simulink model "chapter2_5_2"  (R2022a) (continued)
== system chapter2_5_2/Subsystem5/Subsystem3/Subsystem6 == (continued)
  Add Constant9/1 -> Subsystem11/1(index), Add Constant10/1
  Add Constant3/1 -> Subsystem6/1(index), Add Constant4/1
  Add Constant13/1 -> Add Constant19/1, Subsystem16/1(index)
  Subsystem16/1(Out1) -> Add/18
  [Ff] -> Subsystem19/2(F)

== system chapter2_5_2/Subsystem5/Subsystem3/Subsystem7 ==
  In2 : Inport
  In1 : Inport  Port=2
  Add : Sum  Inputs=++++++++++++++++++++
  Add Constant{,1..16,19..20} (19 alike) : Bias  Bias=1  SaturateOnIntegerOverflow=off
  Subsystem1 : Subsystem
  Subsystem10 : Subsystem
  Subsystem11 : Subsystem
  Subsystem12 : Subsystem
  Subsystem13 : Subsystem
  Subsystem14 : Subsystem
  Subsystem15 : Subsystem
  Subsystem16 : Subsystem
  Subsystem17 : Subsystem
  Subsystem18 : Subsystem
  Subsystem19 : Subsystem
  Subsystem2 : Subsystem
  Subsystem21 : Subsystem
  Subsystem3 : Subsystem
  Subsystem4 : Subsystem
  Subsystem5 : Subsystem
  Subsystem6 : Subsystem
  Subsystem7 : Subsystem
  Subsystem8 : Subsystem
  Subsystem9 : Subsystem
  Out1 : Outport
  wires:
  [Ff] -> Subsystem19/2(F)
  Subsystem16/1(Out1) -> Add/18
  Add Constant13/1 -> Subsystem16/1(index), Add Constant19/1
  Add Constant3/1 -> Add Constant4/1, Subsystem6/1(index)
  Add Constant9/1 -> Add Constant10/1, Subsystem11/1(index)
  Add Constant19/1 -> Subsystem19/1(index), Add Constant20/1
  [Ff] -> Subsystem14/2(F)
  [Ff] -> Subsystem5/2(F)
  Add Constant16/1 -> Add Constant12/1, Subsystem13/1(index)
  Add Constant2/1 -> Add Constant3/1, Subsystem2/1(index)
  [Ff] -> Subsystem2/2(F)
  [Ff] -> Subsystem11/2(F)
  Add Constant/1 -> Add Constant1/1, Subsystem1/1(index)
  Add Constant6/1 -> Add Constant7/1, Subsystem5/1(index)
  [Ff] -> Subsystem7/2(F)
  Add Constant14/1 -> Add Constant15/1, Subsystem12/1(index)
  [Ff] -> Subsystem9/2(F)
  [Ff] -> Subsystem8/2(F)
  [Ff] -> Subsystem16/2(F)
  Add Constant15/1 -> Add Constant16/1, Subsystem15/1(index)
  [Ff] -> Subsystem4/2(F)
  Add Constant20/1 -> Subsystem17/1(index)
  [Ff] -> Subsystem1/2(F)
  [Ff] -> Subsystem18/2(F)
  Add Constant4/1 -> Add Constant5/1, Subsystem4/1(index)
  [Ff] -> Subsystem21/2(F)
  Add Constant5/1 -> Add Constant6/1, Subsystem7/1(index)
  Add Constant12/1 -> Subsystem18/1(index), Add Constant13/1
  In1/1 -> Add Constant/1, Subsystem21/1(index)
  [Ff] -> Subsystem15/2(F)
  Add Constant11/1 -> Add Constant14/1, Subsystem14/1(index)
  Add/1 -> Out1/1
  [Ff] -> Subsystem17/2(F)
  Add Constant1/1 -> Add Constant2/1, Subsystem3/1(index)
  Add Constant8/1 -> Add Constant9/1, Subsystem8/1(index)
  [Ff] -> Subsystem12/2(F)
  Add Constant10/1 -> Add Constant11/1, Subsystem9/1(index)
  [Ff] -> Subsystem6/2(F)
  Add Constant7/1 -> Add Constant8/1, Subsystem10/1(index)
  [Ff] -> Subsystem10/2(F)
  [Ff] -> Subsystem13/2(F)
  [Ff] -> Subsystem3/2(F)
  In2/1 -> [Ff]
  Subsystem19/1(Out1) -> Add/19
  Subsystem17/1(Out1) -> Add/20
  Subsystem18/1(Out1) -> Add/17
  Subsystem13/1(Out1) -> Add/16
  Subsystem15/1(Out1) -> Add/15
  Subsystem10/1(Out1) -> Add/9
  Subsystem8/1(Out1) -> Add/10
  Subsystem11/1(Out1) -> Add/11
  Subsystem12/1(Out1) -> Add/14
  Subsystem14/1(Out1) -> Add/13
  Subsystem9/1(Out1) -> Add/12
  Subsystem21/1(Out1) -> Add/1
  Subsystem1/1(Out1) -> Add/2
  Subsystem3/1(Out1) -> Add/3
  Subsystem2/1(Out1) -> Add/4
  Subsystem6/1(Out1) -> Add/5
  Subsystem5/1(Out1) -> Add/8
  Subsystem7/1(Out1) -> Add/7
  Subsystem4/1(Out1) -> Add/6

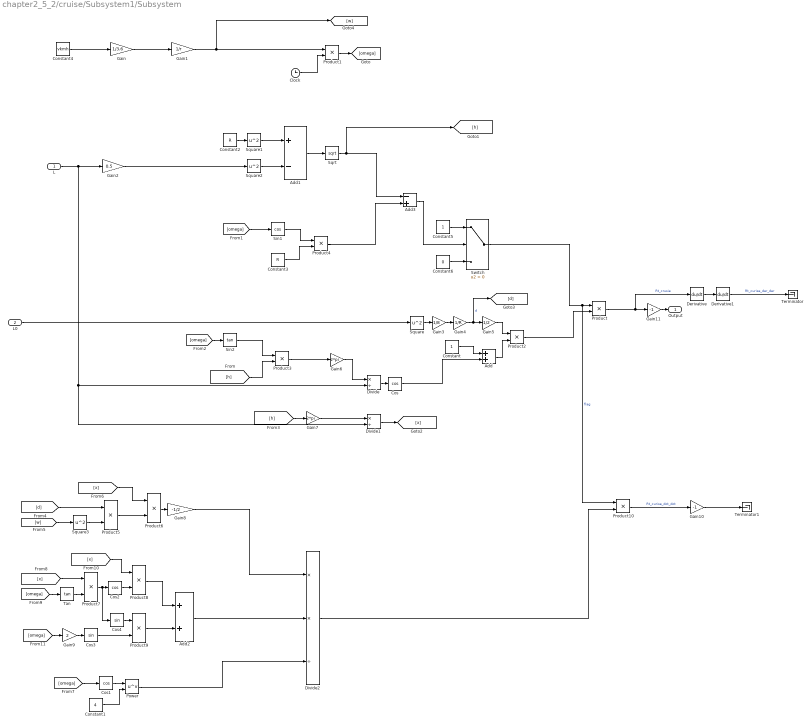
== system chapter2_5_2/cruise/Subsystem1/Subsystem ==
  L : Inport
  L0 : Inport  Port=2
  Add : Sum
  Add1 : Sum  Inputs=+-
  Add2 : Sum
  Add3 : Sum  Inputs=-+
  Clock : Clock
  Constant : Constant
  Constant1 : Constant  Value=4
  Constant2 : Constant  Value=R
  Constant3 : Constant  Value=R
  Constant4 : Constant  Value=vkmh
  Constant5 : Constant
  Constant6 : Constant  Value=0
  Cos : Trigonometry  Operator=cos
  Cos1 : Trigonometry  Operator=cos
  Cos2 : Trigonometry  Operator=cos
  Cos3 : Trigonometry
  Cos4 : Trigonometry
  Derivative : Derivative
  Derivative1 : Derivative
  Divide : Product  Inputs=*/
  Divide1 : Product  Inputs=*/
  Divide2 : Product  Inputs=**/
  Gain : Gain  Gain=1/3.6
  Gain1 : Gain  Gain=1/r
  Gain10 : Gain  Gain=-1
  Gain11 : Gain  Gain=-1
  Gain2 : Gain  Gain=0.5
  Gain3 : Gain  Gain=1/8
  Gain4 : Gain  Gain=1/R
  Gain5 : Gain  Gain=1/2
  Gain6 : Gain  Gain=2*pi
  Gain7 : Gain  Gain=2*pi
  Gain8 : Gain  Gain=-1/2
  Gain9 : Gain  Gain=2
  Power : Math  Operator=pow
  Product{,1..10} (11 alike) : Product
  Sin1 : Trigonometry  Operator=cos
  Sin2 : Trigonometry  Operator=tan
  Sqrt : Sqrt
  Square{,1..3} (4 alike) : Math  Operator=square
  Switch : Switch  Criteria=u2 > Threshold  SaturateOnIntegerOverflow=off  InputSameDT=off
  Tan : Trigonometry  Operator=tan
  Terminator : Terminator
  Terminator1 : Terminator
  Output : Outport
  wires:
  Clock/1 -> Product1/2
  Product1/1 -> [omega]
  Add1/1 -> Sqrt/1
  Gain3/1 -> Gain4/1
  Divide/1 -> Cos/1
  Gain/1 -> Gain1/1
  Product4/1 -> Add3/2
  Constant2/1 -> Square1/1
  Square/1 -> Gain3/1
  Constant6/1 -> Switch/3
  [h] -> Product3/2
  [omega] -> Sin1/1
  Constant3/1 -> Product4/2
  Add3/1 -> Switch/2
  Gain4/1 -> [d], Gain5/1  "d"
  Constant5/1 -> Switch/1
  Square2/1 -> Add1/2
  Switch/1 -> Product10/1, Product/1  "flag"
  Square1/1 -> Add1/1
  Sin1/1 -> Product4/1
  Add/1 -> Product2/2
  Product/1 -> Derivative/1, Gain11/1  "Rt_crusie"
  Sqrt/1 -> [h], Add3/1
  Product2/1 -> Product/2
  Gain1/1 -> Product1/1, [w]
  Product3/1 -> Gain6/1
  Sin2/1 -> Product3/1
  Gain5/1 -> Product2/1
  Cos/1 -> Add/2
  Constant/1 -> Add/1
  L0/1 -> Square/1
  Gain2/1 -> Square2/1
  [omega] -> Sin2/1
  Gain6/1 -> Divide/1
  Constant4/1 -> Gain/1
  L/1 -> Divide/2, Divide1/2, Gain2/1
  [h] -> Gain7/1
  Gain7/1 -> Divide1/1
  Divide1/1 -> [x]
  [w] -> Square3/1
  Square3/1 -> Product5/2
  [d] -> Product5/1
  Product5/1 -> Product6/2
  [x] -> Product6/1
  Product6/1 -> Gain8/1
  [omega] -> Cos1/1
  Cos1/1 -> Power/1
  Constant1/1 -> Power/2
  Power/1 -> Divide2/3
  [omega] -> Tan/1
  Tan/1 -> Product7/2
  [x] -> Product7/1
  Product7/1 -> Cos4/1, Cos2/1
  Cos2/1 -> Product8/2
  [x] -> Product8/1
  Product8/1 -> Add2/1
  [omega] -> Gain9/1
  Gain9/1 -> Cos3/1
  Cos3/1 -> Product9/2
  Cos4/1 -> Product9/1
  Product9/1 -> Add2/2
  Add2/1 -> Divide2/2
  Divide2/1 -> Product10/2
  Product10/1 -> Gain10/1  "Rt_curise_dot_dot"
  Derivative/1 -> Derivative1/1
  Derivative1/1 -> Terminator/1  "Rt_curise_der_der"
  Gain8/1 -> Divide2/1
  Gain10/1 -> Terminator1/1
  Gain11/1 -> Output/1

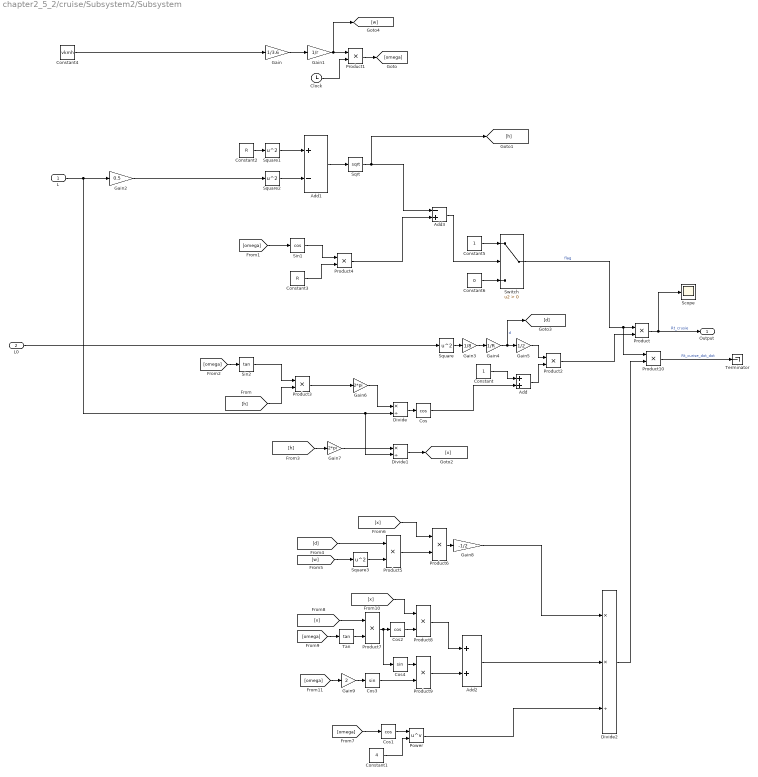
== system chapter2_5_2/cruise/Subsystem2/Subsystem ==
  L : Inport
  L0 : Inport  Port=2
  Add : Sum
  Add1 : Sum  Inputs=+-
  Add2 : Sum
  Add3 : Sum  Inputs=-+
  Clock : Clock
  Constant : Constant
  Constant1 : Constant  Value=4
  Constant2 : Constant  Value=R
  Constant3 : Constant  Value=R
  Constant4 : Constant  Value=vkmh
  Constant5 : Constant
  Constant6 : Constant  Value=0
  Cos : Trigonometry  Operator=cos
  Cos1 : Trigonometry  Operator=cos
  Cos2 : Trigonometry  Operator=cos
  Cos3 : Trigonometry
  Cos4 : Trigonometry
  Divide : Product  Inputs=*/
  Divide1 : Product  Inputs=*/
  Divide2 : Product  Inputs=**/
  Gain : Gain  Gain=1/3.6
  Gain1 : Gain  Gain=1/r
  Gain2 : Gain  Gain=0.5
  Gain3 : Gain  Gain=1/8
  Gain4 : Gain  Gain=1/R
  Gain5 : Gain  Gain=1/2
  Gain6 : Gain  Gain=2*pi
  Gain7 : Gain  Gain=2*pi
  Gain8 : Gain  Gain=-1/2
  Gain9 : Gain  Gain=2
  Power : Math  Operator=pow
  Product{,1..10} (11 alike) : Product
  Scope : Scope
  Sin1 : Trigonometry  Operator=cos
  Sin2 : Trigonometry  Operator=tan
  Sqrt : Sqrt
  Square{,1..3} (4 alike) : Math  Operator=square
  Switch : Switch  Criteria=u2 > Threshold  SaturateOnIntegerOverflow=off  InputSameDT=off
  Tan : Trigonometry  Operator=tan
  Terminator : Terminator
  Output : Outport
  wires:
  Product10/1 -> Terminator/1  "Rt_curise_dot_dot"
  Divide2/1 -> Product10/2
  Add2/1 -> Divide2/2
  Product9/1 -> Add2/2
  Cos4/1 -> Product9/1
  Cos3/1 -> Product9/2
  Gain9/1 -> Cos3/1
  [omega] -> Gain9/1
  Product8/1 -> Add2/1
  [x] -> Product8/1
  Cos2/1 -> Product8/2
  Product7/1 -> Cos2/1, Cos4/1
  [x] -> Product7/1
  Tan/1 -> Product7/2
  [omega] -> Tan/1
  Gain8/1 -> Divide2/1
  Power/1 -> Divide2/3
  Constant1/1 -> Power/2
  Cos1/1 -> Power/1
  [omega] -> Cos1/1
  Product6/1 -> Gain8/1
  [x] -> Product6/1
  Product5/1 -> Product6/2
  [d] -> Product5/1
  Square3/1 -> Product5/2
  [w] -> Square3/1
  Divide1/1 -> [x]
  Gain7/1 -> Divide1/1
  [h] -> Gain7/1
  L/1 -> Gain2/1, Divide/2, Divide1/2
  Constant4/1 -> Gain/1
  Gain6/1 -> Divide/1
  [omega] -> Sin2/1
  Gain2/1 -> Square2/1
  L0/1 -> Square/1
  Constant/1 -> Add/1
  Cos/1 -> Add/2
  Gain5/1 -> Product2/1
  Sin2/1 -> Product3/1
  Product3/1 -> Gain6/1
  Gain1/1 -> Product1/1, [w]
  Product2/1 -> Product/2
  Sqrt/1 -> Add3/1, [h]
  Product/1 -> Scope/1, Output/1  "Rt_crusie"
  Add/1 -> Product2/2
  Sin1/1 -> Product4/1
  Square1/1 -> Add1/1
  Switch/1 -> Product/1, Product10/1  "flag"
  Square2/1 -> Add1/2
  Constant5/1 -> Switch/1
  Gain4/1 -> Gain5/1, [d]  "d"
  Add3/1 -> Switch/2
  Constant3/1 -> Product4/2
  [omega] -> Sin1/1
  [h] -> Product3/2
  Constant6/1 -> Switch/3
  Square/1 -> Gain3/1
  Constant2/1 -> Square1/1
  Product4/1 -> Add3/2
  Gain/1 -> Gain1/1
  Divide/1 -> Cos/1
  Gain3/1 -> Gain4/1
  Add1/1 -> Sqrt/1
  Product1/1 -> [omega]
  Clock/1 -> Product1/2

== system chapter2_5_2/Subsystem5/Subsystem/Subsystem/Subsystem1 ==
  index : Inport
  F : Inport  Port=2
  Add1 : Sum
  Add2 : Sum
  Constant2 : Constant
  Constant6 : Constant
  Constant7 : Constant
  Constant8 : Constant  Value=2
  Demux : Demux  Outputs=2
  Mux : Mux  Inputs=2
  Subsystem : Subsystem
  Subsystem1 : Subsystem
  Subsystem2 : Subsystem
  Subsystem3 : Subsystem
  Out1 : Outport
  wires:
  index/1 -> Subsystem1/1(imod), Subsystem/1(imod)
  Add1/1 -> Mux/1
  Constant2/1 -> Subsystem/2(r)
  Constant6/1 -> Subsystem/3(k)
  Constant8/1 -> Subsystem1/3(k)
  Constant7/1 -> Subsystem1/2(r)
  F/1 -> Demux/1
  Demux/1 -> Subsystem/4(F)
  Demux/2 -> Subsystem1/4(F)
  Subsystem/2(theta_n1) -> Subsystem2/2(theta_n1)
  Subsystem/3(theta_n2) -> Subsystem2/3(theta_n2)
  Subsystem/4(theta_n3) -> Subsystem2/4(theta_n3)
  Subsystem/1(An1) -> Subsystem2/1(An1)
  Subsystem/5(Sn) -> Subsystem2/5(Sn)
  Subsystem/6(static_n) -> Subsystem2/6(static_n)
  Subsystem/7(Omiga_bn) -> Subsystem2/7(Omiga_bn)
  Subsystem/8(Omiga_n) -> Subsystem2/8(Omiga_n)
  Subsystem1/1(An1) -> Subsystem3/1(An1)
  Subsystem1/2(theta_n1) -> Subsystem3/2(theta_n1)
  Subsystem1/3(theta_n2) -> Subsystem3/3(theta_n2)
  Subsystem1/4(theta_n3) -> Subsystem3/4(theta_n3)
  Subsystem1/5(Sn) -> Subsystem3/5(Sn)
  Subsystem1/6(static_n) -> Subsystem3/6(static_n)
  Subsystem1/7(Omiga_bn) -> Subsystem3/7(Omiga_bn)
  Subsystem1/8(Omiga_n) -> Subsystem3/8(Omiga_n)
  Subsystem2/1(Out1) -> Add1/1
  Subsystem3/1(Out1) -> Add1/2
  Add2/1 -> Mux/2
  Subsystem3/2(Out2) -> Add2/2
  Subsystem2/2(Out2) -> Add2/1
  Mux/1 -> Out1/1

== system chapter2_5_2/Subsystem5/Subsystem/Subsystem/Subsystem10 ==
  index : Inport
  F : Inport  Port=2
  Add1 : Sum
  Add2 : Sum
  Constant2 : Constant
  Constant6 : Constant
  Constant7 : Constant
  Constant8 : Constant  Value=2
  Demux : Demux  Outputs=2
  Mux : Mux  Inputs=2
  Subsystem : Subsystem
  Subsystem1 : Subsystem
  Subsystem2 : Subsystem
  Subsystem3 : Subsystem
  Out1 : Outport
  wires:
  index/1 -> Subsystem1/1(imod), Subsystem/1(imod)
  Add1/1 -> Mux/1
  Constant2/1 -> Subsystem/2(r)
  Constant6/1 -> Subsystem/3(k)
  Constant8/1 -> Subsystem1/3(k)
  Constant7/1 -> Subsystem1/2(r)
  F/1 -> Demux/1
  Demux/1 -> Subsystem/4(F)
  Demux/2 -> Subsystem1/4(F)
  Subsystem/2(theta_n1) -> Subsystem2/2(theta_n1)
  Subsystem/3(theta_n2) -> Subsystem2/3(theta_n2)
  Subsystem/4(theta_n3) -> Subsystem2/4(theta_n3)
  Subsystem/1(An1) -> Subsystem2/1(An1)
  Subsystem/5(Sn) -> Subsystem2/5(Sn)
  Subsystem/6(static_n) -> Subsystem2/6(static_n)
  Subsystem/7(Omiga_bn) -> Subsystem2/7(Omiga_bn)
  Subsystem/8(Omiga_n) -> Subsystem2/8(Omiga_n)
  Subsystem1/1(An1) -> Subsystem3/1(An1)
  Subsystem1/2(theta_n1) -> Subsystem3/2(theta_n1)
  Subsystem1/3(theta_n2) -> Subsystem3/3(theta_n2)
  Subsystem1/4(theta_n3) -> Subsystem3/4(theta_n3)
  Subsystem1/5(Sn) -> Subsystem3/5(Sn)
  Subsystem1/6(static_n) -> Subsystem3/6(static_n)
  Subsystem1/7(Omiga_bn) -> Subsystem3/7(Omiga_bn)
  Subsystem1/8(Omiga_n) -> Subsystem3/8(Omiga_n)
  Subsystem2/1(Out1) -> Add1/1
  Subsystem3/1(Out1) -> Add1/2
  Add2/1 -> Mux/2
  Subsystem3/2(Out2) -> Add2/2
  Subsystem2/2(Out2) -> Add2/1
  Mux/1 -> Out1/1

== system chapter2_5_2/Subsystem5/Subsystem/Subsystem/Subsystem11 ==
  index : Inport
  F : Inport  Port=2
  Add1 : Sum
  Add2 : Sum
  Constant2 : Constant
  Constant6 : Constant
  Constant7 : Constant
  Constant8 : Constant  Value=2
  Demux : Demux  Outputs=2
  Mux : Mux  Inputs=2
  Subsystem : Subsystem
  Subsystem1 : Subsystem
  Subsystem2 : Subsystem
  Subsystem3 : Subsystem
  Out1 : Outport
  wires:
  Mux/1 -> Out1/1
  Subsystem2/2(Out2) -> Add2/1
  Subsystem3/2(Out2) -> Add2/2
  Add2/1 -> Mux/2
  Subsystem3/1(Out1) -> Add1/2
  Subsystem2/1(Out1) -> Add1/1
  Subsystem1/8(Omiga_n) -> Subsystem3/8(Omiga_n)
  Subsystem1/7(Omiga_bn) -> Subsystem3/7(Omiga_bn)
  Subsystem1/6(static_n) -> Subsystem3/6(static_n)
  Subsystem1/5(Sn) -> Subsystem3/5(Sn)
  Subsystem1/4(theta_n3) -> Subsystem3/4(theta_n3)
  Subsystem1/3(theta_n2) -> Subsystem3/3(theta_n2)
  Subsystem1/2(theta_n1) -> Subsystem3/2(theta_n1)
  Subsystem1/1(An1) -> Subsystem3/1(An1)
  Subsystem/8(Omiga_n) -> Subsystem2/8(Omiga_n)
  Subsystem/7(Omiga_bn) -> Subsystem2/7(Omiga_bn)
  Subsystem/6(static_n) -> Subsystem2/6(static_n)
  Subsystem/5(Sn) -> Subsystem2/5(Sn)
  Subsystem/1(An1) -> Subsystem2/1(An1)
  Subsystem/4(theta_n3) -> Subsystem2/4(theta_n3)
  Subsystem/3(theta_n2) -> Subsystem2/3(theta_n2)
  Subsystem/2(theta_n1) -> Subsystem2/2(theta_n1)
  Demux/2 -> Subsystem1/4(F)
  Demux/1 -> Subsystem/4(F)
  F/1 -> Demux/1
  Constant7/1 -> Subsystem1/2(r)
  Constant8/1 -> Subsystem1/3(k)
  Constant6/1 -> Subsystem/3(k)
  Constant2/1 -> Subsystem/2(r)
  Add1/1 -> Mux/1
  index/1 -> Subsystem/1(imod), Subsystem1/1(imod)

== system chapter2_5_2/Subsystem5/Subsystem/Subsystem/Subsystem12 ==
  index : Inport
  F : Inport  Port=2
  Add1 : Sum
  Add2 : Sum
  Constant2 : Constant
  Constant6 : Constant
  Constant7 : Constant
  Constant8 : Constant  Value=2
  Demux : Demux  Outputs=2
  Mux : Mux  Inputs=2
  Subsystem : Subsystem
  Subsystem1 : Subsystem
  Subsystem2 : Subsystem
  Subsystem3 : Subsystem
  Out1 : Outport
  wires:
  index/1 -> Subsystem1/1(imod), Subsystem/1(imod)
  Add1/1 -> Mux/1
  Constant2/1 -> Subsystem/2(r)
  Constant6/1 -> Subsystem/3(k)
  Constant8/1 -> Subsystem1/3(k)
  Constant7/1 -> Subsystem1/2(r)
  F/1 -> Demux/1
  Demux/1 -> Subsystem/4(F)
  Demux/2 -> Subsystem1/4(F)
  Subsystem/2(theta_n1) -> Subsystem2/2(theta_n1)
  Subsystem/3(theta_n2) -> Subsystem2/3(theta_n2)
  Subsystem/4(theta_n3) -> Subsystem2/4(theta_n3)
  Subsystem/1(An1) -> Subsystem2/1(An1)
  Subsystem/5(Sn) -> Subsystem2/5(Sn)
  Subsystem/6(static_n) -> Subsystem2/6(static_n)
  Subsystem/7(Omiga_bn) -> Subsystem2/7(Omiga_bn)
  Subsystem/8(Omiga_n) -> Subsystem2/8(Omiga_n)
  Subsystem1/1(An1) -> Subsystem3/1(An1)
  Subsystem1/2(theta_n1) -> Subsystem3/2(theta_n1)
  Subsystem1/3(theta_n2) -> Subsystem3/3(theta_n2)
  Subsystem1/4(theta_n3) -> Subsystem3/4(theta_n3)
  Subsystem1/5(Sn) -> Subsystem3/5(Sn)
  Subsystem1/6(static_n) -> Subsystem3/6(static_n)
  Subsystem1/7(Omiga_bn) -> Subsystem3/7(Omiga_bn)
  Subsystem1/8(Omiga_n) -> Subsystem3/8(Omiga_n)
  Subsystem2/1(Out1) -> Add1/1
  Subsystem3/1(Out1) -> Add1/2
  Add2/1 -> Mux/2
  Subsystem3/2(Out2) -> Add2/2
  Subsystem2/2(Out2) -> Add2/1
  Mux/1 -> Out1/1

== system chapter2_5_2/Subsystem5/Subsystem/Subsystem/Subsystem13 ==
  index : Inport
  F : Inport  Port=2
  Add1 : Sum
  Add2 : Sum
  Constant2 : Constant
  Constant6 : Constant
  Constant7 : Constant
  Constant8 : Constant  Value=2
  Demux : Demux  Outputs=2
  Mux : Mux  Inputs=2
  Subsystem : Subsystem
  Subsystem1 : Subsystem
  Subsystem2 : Subsystem
  Subsystem3 : Subsystem
  Out1 : Outport
  wires:
  Mux/1 -> Out1/1
  Subsystem2/2(Out2) -> Add2/1
  Subsystem3/2(Out2) -> Add2/2
  Add2/1 -> Mux/2
  Subsystem3/1(Out1) -> Add1/2
  Subsystem2/1(Out1) -> Add1/1
  Subsystem1/8(Omiga_n) -> Subsystem3/8(Omiga_n)
  Subsystem1/7(Omiga_bn) -> Subsystem3/7(Omiga_bn)
  Subsystem1/6(static_n) -> Subsystem3/6(static_n)
  Subsystem1/5(Sn) -> Subsystem3/5(Sn)
  Subsystem1/4(theta_n3) -> Subsystem3/4(theta_n3)
  Subsystem1/3(theta_n2) -> Subsystem3/3(theta_n2)
  Subsystem1/2(theta_n1) -> Subsystem3/2(theta_n1)
  Subsystem1/1(An1) -> Subsystem3/1(An1)
  Subsystem/8(Omiga_n) -> Subsystem2/8(Omiga_n)
  Subsystem/7(Omiga_bn) -> Subsystem2/7(Omiga_bn)
  Subsystem/6(static_n) -> Subsystem2/6(static_n)
  Subsystem/5(Sn) -> Subsystem2/5(Sn)
  Subsystem/1(An1) -> Subsystem2/1(An1)
  Subsystem/4(theta_n3) -> Subsystem2/4(theta_n3)
  Subsystem/3(theta_n2) -> Subsystem2/3(theta_n2)
  Subsystem/2(theta_n1) -> Subsystem2/2(theta_n1)
  Demux/2 -> Subsystem1/4(F)
  Demux/1 -> Subsystem/4(F)
  F/1 -> Demux/1
  Constant7/1 -> Subsystem1/2(r)
  Constant8/1 -> Subsystem1/3(k)
  Constant6/1 -> Subsystem/3(k)
  Constant2/1 -> Subsystem/2(r)
  Add1/1 -> Mux/1
  index/1 -> Subsystem/1(imod), Subsystem1/1(imod)

== system chapter2_5_2/Subsystem5/Subsystem/Subsystem/Subsystem14 ==
  index : Inport
  F : Inport  Port=2
  Add1 : Sum
  Add2 : Sum
  Constant2 : Constant
  Constant6 : Constant
  Constant7 : Constant
  Constant8 : Constant  Value=2
  Demux : Demux  Outputs=2
  Mux : Mux  Inputs=2
  Subsystem : Subsystem
  Subsystem1 : Subsystem
  Subsystem2 : Subsystem
  Subsystem3 : Subsystem
  Out1 : Outport
  wires:
  index/1 -> Subsystem1/1(imod), Subsystem/1(imod)
  Add1/1 -> Mux/1
  Constant2/1 -> Subsystem/2(r)
  Constant6/1 -> Subsystem/3(k)
  Constant8/1 -> Subsystem1/3(k)
  Constant7/1 -> Subsystem1/2(r)
  F/1 -> Demux/1
  Demux/1 -> Subsystem/4(F)
  Demux/2 -> Subsystem1/4(F)
  Subsystem/2(theta_n1) -> Subsystem2/2(theta_n1)
  Subsystem/3(theta_n2) -> Subsystem2/3(theta_n2)
  Subsystem/4(theta_n3) -> Subsystem2/4(theta_n3)
  Subsystem/1(An1) -> Subsystem2/1(An1)
  Subsystem/5(Sn) -> Subsystem2/5(Sn)
  Subsystem/6(static_n) -> Subsystem2/6(static_n)
  Subsystem/7(Omiga_bn) -> Subsystem2/7(Omiga_bn)
  Subsystem/8(Omiga_n) -> Subsystem2/8(Omiga_n)
  Subsystem1/1(An1) -> Subsystem3/1(An1)
  Subsystem1/2(theta_n1) -> Subsystem3/2(theta_n1)
  Subsystem1/3(theta_n2) -> Subsystem3/3(theta_n2)
  Subsystem1/4(theta_n3) -> Subsystem3/4(theta_n3)
  Subsystem1/5(Sn) -> Subsystem3/5(Sn)
  Subsystem1/6(static_n) -> Subsystem3/6(static_n)
  Subsystem1/7(Omiga_bn) -> Subsystem3/7(Omiga_bn)
  Subsystem1/8(Omiga_n) -> Subsystem3/8(Omiga_n)
  Subsystem2/1(Out1) -> Add1/1
  Subsystem3/1(Out1) -> Add1/2
  Add2/1 -> Mux/2
  Subsystem3/2(Out2) -> Add2/2
  Subsystem2/2(Out2) -> Add2/1
  Mux/1 -> Out1/1

== system chapter2_5_2/Subsystem5/Subsystem/Subsystem/Subsystem15 ==
  index : Inport
  F : Inport  Port=2
  Add1 : Sum
  Add2 : Sum
  Constant2 : Constant
  Constant6 : Constant
  Constant7 : Constant
  Constant8 : Constant  Value=2
  Demux : Demux  Outputs=2
  Mux : Mux  Inputs=2
  Subsystem : Subsystem
  Subsystem1 : Subsystem
  Subsystem2 : Subsystem
  Subsystem3 : Subsystem
  Out1 : Outport
  wires:
  index/1 -> Subsystem1/1(imod), Subsystem/1(imod)
  Add1/1 -> Mux/1
  Constant2/1 -> Subsystem/2(r)
  Constant6/1 -> Subsystem/3(k)
  Constant8/1 -> Subsystem1/3(k)
  Constant7/1 -> Subsystem1/2(r)
  F/1 -> Demux/1
  Demux/1 -> Subsystem/4(F)
  Demux/2 -> Subsystem1/4(F)
  Subsystem/2(theta_n1) -> Subsystem2/2(theta_n1)
  Subsystem/3(theta_n2) -> Subsystem2/3(theta_n2)
  Subsystem/4(theta_n3) -> Subsystem2/4(theta_n3)
  Subsystem/1(An1) -> Subsystem2/1(An1)
  Subsystem/5(Sn) -> Subsystem2/5(Sn)
  Subsystem/6(static_n) -> Subsystem2/6(static_n)
  Subsystem/7(Omiga_bn) -> Subsystem2/7(Omiga_bn)
  Subsystem/8(Omiga_n) -> Subsystem2/8(Omiga_n)
  Subsystem1/1(An1) -> Subsystem3/1(An1)
  Subsystem1/2(theta_n1) -> Subsystem3/2(theta_n1)
  Subsystem1/3(theta_n2) -> Subsystem3/3(theta_n2)
  Subsystem1/4(theta_n3) -> Subsystem3/4(theta_n3)
  Subsystem1/5(Sn) -> Subsystem3/5(Sn)
  Subsystem1/6(static_n) -> Subsystem3/6(static_n)
  Subsystem1/7(Omiga_bn) -> Subsystem3/7(Omiga_bn)
  Subsystem1/8(Omiga_n) -> Subsystem3/8(Omiga_n)
  Subsystem2/1(Out1) -> Add1/1
  Subsystem3/1(Out1) -> Add1/2
  Add2/1 -> Mux/2
  Subsystem3/2(Out2) -> Add2/2
  Subsystem2/2(Out2) -> Add2/1
  Mux/1 -> Out1/1

== system chapter2_5_2/Subsystem5/Subsystem/Subsystem/Subsystem16 ==
  index : Inport
  F : Inport  Port=2
  Add1 : Sum
  Add2 : Sum
  Constant2 : Constant
  Constant6 : Constant
  Constant7 : Constant
  Constant8 : Constant  Value=2
  Demux : Demux  Outputs=2
  Mux : Mux  Inputs=2
  Subsystem : Subsystem
  Subsystem1 : Subsystem
  Subsystem2 : Subsystem
  Subsystem3 : Subsystem
  Out1 : Outport
  wires:
  Mux/1 -> Out1/1
  Subsystem2/2(Out2) -> Add2/1
  Subsystem3/2(Out2) -> Add2/2
  Add2/1 -> Mux/2
  Subsystem3/1(Out1) -> Add1/2
  Subsystem2/1(Out1) -> Add1/1
  Subsystem1/8(Omiga_n) -> Subsystem3/8(Omiga_n)
  Subsystem1/7(Omiga_bn) -> Subsystem3/7(Omiga_bn)
  Subsystem1/6(static_n) -> Subsystem3/6(static_n)
  Subsystem1/5(Sn) -> Subsystem3/5(Sn)
  Subsystem1/4(theta_n3) -> Subsystem3/4(theta_n3)
  Subsystem1/3(theta_n2) -> Subsystem3/3(theta_n2)
  Subsystem1/2(theta_n1) -> Subsystem3/2(theta_n1)
  Subsystem1/1(An1) -> Subsystem3/1(An1)
  Subsystem/8(Omiga_n) -> Subsystem2/8(Omiga_n)
  Subsystem/7(Omiga_bn) -> Subsystem2/7(Omiga_bn)
  Subsystem/6(static_n) -> Subsystem2/6(static_n)
  Subsystem/5(Sn) -> Subsystem2/5(Sn)
  Subsystem/1(An1) -> Subsystem2/1(An1)
  Subsystem/4(theta_n3) -> Subsystem2/4(theta_n3)
  Subsystem/3(theta_n2) -> Subsystem2/3(theta_n2)
  Subsystem/2(theta_n1) -> Subsystem2/2(theta_n1)
  Demux/2 -> Subsystem1/4(F)
  Demux/1 -> Subsystem/4(F)
  F/1 -> Demux/1
  Constant7/1 -> Subsystem1/2(r)
  Constant8/1 -> Subsystem1/3(k)
  Constant6/1 -> Subsystem/3(k)
  Constant2/1 -> Subsystem/2(r)
  Add1/1 -> Mux/1
  index/1 -> Subsystem/1(imod), Subsystem1/1(imod)

== system chapter2_5_2/Subsystem5/Subsystem/Subsystem/Subsystem17 ==
  index : Inport
  F : Inport  Port=2
  Add1 : Sum
  Add2 : Sum
  Constant2 : Constant
  Constant6 : Constant
  Constant7 : Constant
  Constant8 : Constant  Value=2
  Demux : Demux  Outputs=2
  Mux : Mux  Inputs=2
  Subsystem : Subsystem
  Subsystem1 : Subsystem
  Subsystem2 : Subsystem
  Subsystem3 : Subsystem
  Out1 : Outport
  wires:
  Mux/1 -> Out1/1
  Subsystem2/2(Out2) -> Add2/1
  Subsystem3/2(Out2) -> Add2/2
  Add2/1 -> Mux/2
  Subsystem3/1(Out1) -> Add1/2
  Subsystem2/1(Out1) -> Add1/1
  Subsystem1/8(Omiga_n) -> Subsystem3/8(Omiga_n)
  Subsystem1/7(Omiga_bn) -> Subsystem3/7(Omiga_bn)
  Subsystem1/6(static_n) -> Subsystem3/6(static_n)
  Subsystem1/5(Sn) -> Subsystem3/5(Sn)
  Subsystem1/4(theta_n3) -> Subsystem3/4(theta_n3)
  Subsystem1/3(theta_n2) -> Subsystem3/3(theta_n2)
  Subsystem1/2(theta_n1) -> Subsystem3/2(theta_n1)
  Subsystem1/1(An1) -> Subsystem3/1(An1)
  Subsystem/8(Omiga_n) -> Subsystem2/8(Omiga_n)
  Subsystem/7(Omiga_bn) -> Subsystem2/7(Omiga_bn)
  Subsystem/6(static_n) -> Subsystem2/6(static_n)
  Subsystem/5(Sn) -> Subsystem2/5(Sn)
  Subsystem/1(An1) -> Subsystem2/1(An1)
  Subsystem/4(theta_n3) -> Subsystem2/4(theta_n3)
  Subsystem/3(theta_n2) -> Subsystem2/3(theta_n2)
  Subsystem/2(theta_n1) -> Subsystem2/2(theta_n1)
  Demux/2 -> Subsystem1/4(F)
  Demux/1 -> Subsystem/4(F)
  F/1 -> Demux/1
  Constant7/1 -> Subsystem1/2(r)
  Constant8/1 -> Subsystem1/3(k)
  Constant6/1 -> Subsystem/3(k)
  Constant2/1 -> Subsystem/2(r)
  Add1/1 -> Mux/1
  index/1 -> Subsystem/1(imod), Subsystem1/1(imod)
